simulink model "DoorWinBltSigMgr"  (R2022b)
settings: Solver=FixedStepDiscrete  StartTime=0.0  StopTime=10.0  FixedStep=0.1  RelTol=1e-3
settings Model: Creator=blue.ge  LastModifiedBy=blue.ge
settings DataIOCC: SaveFormat=StructureWithTime
settings OptimizationCC: DefaultParameterBehavior=Inlined  EnhancedBackFolding=on  PassReuseOutputArgsAs=Individual arguments  ZeroExternalMemoryAtStartup=off  ZeroInternalMemoryAtStartup=off  OptimizeBlockOrder=speed  DifferentSizesBufferReuse=on  OptimizationCustomize=off  EfficientTunableParamExpr=on
settings DebuggingCC: ParameterTunabilityLossMsg=error
settings ModelReferenceCC: ModelReferenceNumInstancesAllowed=Single
settings SFSimCC: MATLABDynamicMemAlloc=off
settings RTWCC: Description=AUTOSAR  SystemTargetFile=autosar.tlc  GenCodeOnly=on  TemplateMakefile=ert_default_tmf
settings CodeAppCC: ForceParamTrailComments=on  IgnoreCustomStorageClasses=off  OperatorAnnotations=on  SimulinkDataObjDesc=on  SFDataObjDesc=on  InsertBlockDesc=on
settings STFCustomTargetCC: TargetLangStandard=C99 (ISO)  MultiwordLength=256  ParMdlRefBuildCompliant=on  CompOptLevelCompliant=on  IncludeMdlTerminateFcn=off  CombineOutputUpdateFcns=on  SuppressErrorStatus=on  ERTCustomFileBanners=on  SupportNonFinite=off  SupportComplex=off  SupportContinuousTime=off  SupportNonInlinedSFcns=off  RemoveResetFunc=on  MATLABClassNameForMDSCustomization=Simulink.SoftwareTarget.ERTCustomization  AutosarCompliant=on  UseToolchainInfoCompliant=on  LookupTableObjectStructAxisOrder=2,1,3,4,...  LUTObjectStructOrderEvenSpacing=Size,Table,Breakpoints  SystemTargetFile=autosar.tlc
settings ERTTargetCC: TargetLangStandard=C99 (ISO)  ConcurrentExecutionCompliant=off  IncludeMdlTerminateFcn=off  SuppressErrorStatus=on  SupportNonFinite=off  SupportComplex=off  ModelStepFunctionPrototypeControlCompliant=off  CPPClassGenCompliant=off  AutosarCompliant=on  LookupTableObjectStructAxisOrder=2,1,3,4,...  LUTObjectStructOrderEvenSpacing=Size,Table,Breakpoints  InlinedParameterPlacement=NonHierarchical  ERTCustomFileTemplate=example_file_process.tlc

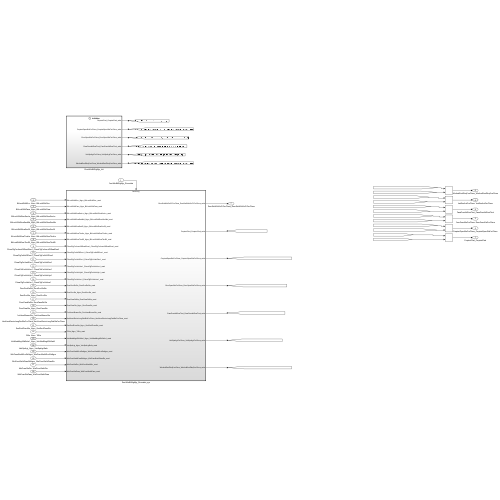
[thumbnail: Simulink's stored preview of the top-level diagram]
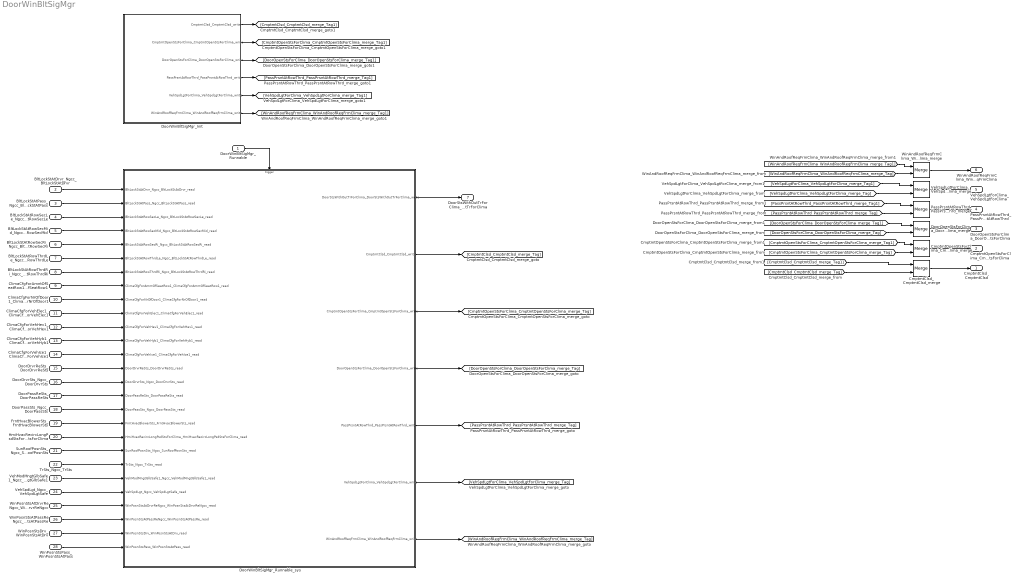
== system DoorWinBltSigMgr ==
  DoorWinBltSigMgr_Runnable : Inport  SampleTime=0.1  OutputFunctionCall=on
  BltLockStAtDrvr_Ngcc_BltLockStAtDrvr : Inport  Port=2  OutDataTypeStr=Bus: BltLockStFrnt  PortDimensions=1  BusOutputAsStruct=on
  BltLockStAtPass_Ngcc_BltLockStAtPass : Inport  Port=3  OutDataTypeStr=Bus: BltLockStFrnt  PortDimensions=1  BusOutputAsStruct=on
  BltLockStAtRowSecLe_Ngcc_BltLockStAtRowSecLe : Inport  Port=4  OutDataTypeStr=Bus: BltLockStRe  PortDimensions=1  BusOutputAsStruct=on
  BltLockStAtRowSecMid_Ngcc_BltLockStAtRowSecMid : Inport  Port=5  OutDataTypeStr=Bus: BltLockStRe  PortDimensions=1  BusOutputAsStruct=on
  BltLockStAtRowSecRi_Ngcc_BltLockStAtRowSecRi : Inport  Port=6  OutDataTypeStr=Bus: BltLockStRe  PortDimensions=1  BusOutputAsStruct=on
  BltLockStAtRowThrdLe_Ngcc_BltLockStAtRowThrdLe : Inport  Port=7  OutDataTypeStr=Bus: BltLockStRe  PortDimensions=1  BusOutputAsStruct=on
  BltLockStAtRowThrdRi_Ngcc_BltLockStAtRowThrdRi : Inport  Port=8  OutDataTypeStr=Bus: BltLockStRe  PortDimensions=1  BusOutputAsStruct=on
  ClimaCfgForAmntOfSeatRow1_ClimaCfgForAmntOfSeatRow1 : Inport  Port=9  OutDataTypeStr=Enum: ClimaCfgForAmntOfSeatRow1  PortDimensions=1
  ClimaCfgForNrOfDoor1_ClimaCfgForNrOfDoor1 : Inport  Port=10  OutDataTypeStr=Enum: ClimaCfgForNrOfDoor1  PortDimensions=1
  ClimaCfgForVehElec1_ClimaCfgForVehElec1 : Inport  Port=11  OutDataTypeStr=Boolean  PortDimensions=1
  ClimaCfgForVehHev1_ClimaCfgForVehHev1 : Inport  Port=12  OutDataTypeStr=Boolean  PortDimensions=1
  ClimaCfgForVehHyb1_ClimaCfgForVehHyb1 : Inport  Port=13  OutDataTypeStr=Boolean  PortDimensions=1
  ClimaCfgForVehIce1_ClimaCfgForVehIce1 : Inport  Port=14  OutDataTypeStr=Boolean  PortDimensions=1
  DoorDrvrReSts_DoorDrvrReSts : Inport  Port=15  OutDataTypeStr=Enum: DoorSts2  PortDimensions=1
  DoorDrvrSts_Ngcc_DoorDrvrSts : Inport  Port=16  OutDataTypeStr=Enum: DoorSts2  PortDimensions=1
  DoorPassReSts_DoorPassReSts : Inport  Port=17  OutDataTypeStr=Enum: DoorSts2  PortDimensions=1
  DoorPassSts_Ngcc_DoorPassSts : Inport  Port=18  OutDataTypeStr=Enum: DoorSts2  PortDimensions=1
  FrntHvacBlowerSts_FrntHvacBlowerSts : Inport  Port=19  OutDataTypeStr=Enum: HvacFanSts  PortDimensions=1
  HmiHvacRecircLongPsdStsForClima_HmiHvacRecircLongPsdStsForClima : Inport  Port=20  OutDataTypeStr=Enum: OnOff1  PortDimensions=1
  SunRoofPosnSts_Ngcc_SunRoofPosnSts : Inport  Port=21  OutDataTypeStr=Enum: WinAndRoofAndCurtPosnTyp  PortDimensions=1
  TrSts_Ngcc_TrSts : Inport  Port=22  OutDataTypeStr=Enum: DoorSts2  PortDimensions=1
  VehModMngtGlbSafe1_Ngcc_VehModMngtGlbSafe1 : Inport  Port=23  OutDataTypeStr=Bus: VehModMngtGlbSafe1  PortDimensions=1  BusOutputAsStruct=on
  VehSpdLgt_Ngcc_VehSpdLgtSafe : Inport  Port=24  OutDataTypeStr=Bus: VehSpdLgtSafe1  PortDimensions=1  BusOutputAsStruct=on
  WinPosnStsAtDrvrReNgcc_WinPosnStsAtDrvrReNgcc : Inport  Port=25  OutDataTypeStr=Enum: WinAndRoofAndCurtPosnTyp  PortDimensions=1
  WinPosnStsAtPassReNgcc_WinPosnStsAtPassRe : Inport  Port=26  OutDataTypeStr=Enum: WinAndRoofAndCurtPosnTyp  PortDimensions=1
  WinPosnStsDrv_WinPosnStsAtDrv : Inport  Port=27  OutDataTypeStr=Enum: WinAndRoofAndCurtPosnTyp  PortDimensions=1
  WinPosnStsPass_WinPosnStsAtPass : Inport  Port=28  OutDataTypeStr=Enum: WinAndRoofAndCurtPosnTyp  PortDimensions=1
  CmptmtClsd_CmptmtClsd_merge : Merge
  CmptmtOpenStsForClima_CmptmtOpenStsForClima_merge : Merge
  DoorOpenStsForClima_DoorOpenStsForClima_merge : Merge
  DoorWinBltSigMgr_Init : Subsystem
  DoorWinBltSigMgr_Runnable_sys : Subsystem
  PassPrsntAtRowThrd_PassPrsntAtRowThrd_merge : Merge
  VehSpdLgtForClima_VehSpdLgtForClima_merge : Merge
  WinAndRoofReqFrmClima_WinAndRoofReqFrmClima_merge : Merge
  CmptmtClsd_CmptmtClsd : Outport  OutDataTypeStr=Boolean  PortDimensions=1
  CmptmtOpenStsForClima_CmptmtOpenStsForClima : Outport  Port=2  OutDataTypeStr=Boolean  PortDimensions=1
  DoorOpenStsForClima_DoorOpenStsForClima : Outport  Port=3  OutDataTypeStr=Boolean  PortDimensions=1
  PassPrsntAtRowThrd_PassPrsntAtRowThrd : Outport  Port=4  OutDataTypeStr=Boolean  PortDimensions=1
  VehSpdLgtForClima_VehSpdLgtForClima : Outport  Port=5  OutDataTypeStr=Bus: VehSpdLgtForClima  PortDimensions=1  BusOutputAsStruct=on
  WinAndRoofReqFrmClima_WinAndRoofReqFrmClima : Outport  Port=6  OutDataTypeStr=Enum: WinOpenClsGlbSts  PortDimensions=1
  DoorStsWithOutTrForClima_DoorStsWithOutTrForClima : Outport  Port=7  OutDataTypeStr=Boolean  PortDimensions=1
  wires:
  BltLockStAtDrvr_Ngcc_BltLockStAtDrvr/1 -> DoorWinBltSigMgr_Runnable_sys/1(BltLockStAtDrvr_Ngcc_BltLockStAtDrvr_read)
  BltLockStAtPass_Ngcc_BltLockStAtPass/1 -> DoorWinBltSigMgr_Runnable_sys/2(BltLockStAtPass_Ngcc_BltLockStAtPass_read)
  BltLockStAtRowSecLe_Ngcc_BltLockStAtRowSecLe/1 -> DoorWinBltSigMgr_Runnable_sys/3(BltLockStAtRowSecLe_Ngcc_BltLockStAtRowSecLe_read)
  BltLockStAtRowSecMid_Ngcc_BltLockStAtRowSecMid/1 -> DoorWinBltSigMgr_Runnable_sys/4(BltLockStAtRowSecMid_Ngcc_BltLockStAtRowSecMid_read)
  BltLockStAtRowSecRi_Ngcc_BltLockStAtRowSecRi/1 -> DoorWinBltSigMgr_Runnable_sys/5(BltLockStAtRowSecRi_Ngcc_BltLockStAtRowSecRi_read)
  BltLockStAtRowThrdLe_Ngcc_BltLockStAtRowThrdLe/1 -> DoorWinBltSigMgr_Runnable_sys/6(BltLockStAtRowThrdLe_Ngcc_BltLockStAtRowThrdLe_read)
  BltLockStAtRowThrdRi_Ngcc_BltLockStAtRowThrdRi/1 -> DoorWinBltSigMgr_Runnable_sys/7(BltLockStAtRowThrdRi_Ngcc_BltLockStAtRowThrdRi_read)
  ClimaCfgForAmntOfSeatRow1_ClimaCfgForAmntOfSeatRow1/1 -> DoorWinBltSigMgr_Runnable_sys/8(ClimaCfgForAmntOfSeatRow1_ClimaCfgForAmntOfSeatRow1_read)
  ClimaCfgForNrOfDoor1_ClimaCfgForNrOfDoor1/1 -> DoorWinBltSigMgr_Runnable_sys/9(ClimaCfgForNrOfDoor1_ClimaCfgForNrOfDoor1_read)
  ClimaCfgForVehElec1_ClimaCfgForVehElec1/1 -> DoorWinBltSigMgr_Runnable_sys/10(ClimaCfgForVehElec1_ClimaCfgForVehElec1_read)
  ClimaCfgForVehHev1_ClimaCfgForVehHev1/1 -> DoorWinBltSigMgr_Runnable_sys/11(ClimaCfgForVehHev1_ClimaCfgForVehHev1_read)
  ClimaCfgForVehHyb1_ClimaCfgForVehHyb1/1 -> DoorWinBltSigMgr_Runnable_sys/12(ClimaCfgForVehHyb1_ClimaCfgForVehHyb1_read)
  ClimaCfgForVehIce1_ClimaCfgForVehIce1/1 -> DoorWinBltSigMgr_Runnable_sys/13(ClimaCfgForVehIce1_ClimaCfgForVehIce1_read)
  DoorDrvrReSts_DoorDrvrReSts/1 -> DoorWinBltSigMgr_Runnable_sys/14(DoorDrvrReSts_DoorDrvrReSts_read)
  DoorDrvrSts_Ngcc_DoorDrvrSts/1 -> DoorWinBltSigMgr_Runnable_sys/15(DoorDrvrSts_Ngcc_DoorDrvrSts_read)
  DoorPassReSts_DoorPassReSts/1 -> DoorWinBltSigMgr_Runnable_sys/16(DoorPassReSts_DoorPassReSts_read)
  DoorPassSts_Ngcc_DoorPassSts/1 -> DoorWinBltSigMgr_Runnable_sys/17(DoorPassSts_Ngcc_DoorPassSts_read)
  FrntHvacBlowerSts_FrntHvacBlowerSts/1 -> DoorWinBltSigMgr_Runnable_sys/18(FrntHvacBlowerSts_FrntHvacBlowerSts_read)
  HmiHvacRecircLongPsdStsForClima_HmiHvacRecircLongPsdStsForClima/1 -> DoorWinBltSigMgr_Runnable_sys/19(HmiHvacRecircLongPsdStsForClima_HmiHvacRecircLongPsdStsForClima_read)
  SunRoofPosnSts_Ngcc_SunRoofPosnSts/1 -> DoorWinBltSigMgr_Runnable_sys/20(SunRoofPosnSts_Ngcc_SunRoofPosnSts_read)
  TrSts_Ngcc_TrSts/1 -> DoorWinBltSigMgr_Runnable_sys/21(TrSts_Ngcc_TrSts_read)
  VehModMngtGlbSafe1_Ngcc_VehModMngtGlbSafe1/1 -> DoorWinBltSigMgr_Runnable_sys/22(VehModMngtGlbSafe1_Ngcc_VehModMngtGlbSafe1_read)
  VehSpdLgt_Ngcc_VehSpdLgtSafe/1 -> DoorWinBltSigMgr_Runnable_sys/23(VehSpdLgt_Ngcc_VehSpdLgtSafe_read)
  WinPosnStsAtDrvrReNgcc_WinPosnStsAtDrvrReNgcc/1 -> DoorWinBltSigMgr_Runnable_sys/24(WinPosnStsAtDrvrReNgcc_WinPosnStsAtDrvrReNgcc_read)
  WinPosnStsAtPassReNgcc_WinPosnStsAtPassRe/1 -> DoorWinBltSigMgr_Runnable_sys/25(WinPosnStsAtPassReNgcc_WinPosnStsAtPassRe_read)
  WinPosnStsDrv_WinPosnStsAtDrv/1 -> DoorWinBltSigMgr_Runnable_sys/26(WinPosnStsDrv_WinPosnStsAtDrv_read)
  WinPosnStsPass_WinPosnStsAtPass/1 -> DoorWinBltSigMgr_Runnable_sys/27(WinPosnStsPass_WinPosnStsAtPass_read)
  DoorWinBltSigMgr_Runnable_sys/1(DoorStsWithOutTrForClima_DoorStsWithOutTrForClima_write) -> DoorStsWithOutTrForClima_DoorStsWithOutTrForClima/1
  CmptmtClsd_CmptmtClsd_merge/1 -> CmptmtClsd_CmptmtClsd/1
  CmptmtOpenStsForClima_CmptmtOpenStsForClima_merge/1 -> CmptmtOpenStsForClima_CmptmtOpenStsForClima/1
  DoorOpenStsForClima_DoorOpenStsForClima_merge/1 -> DoorOpenStsForClima_DoorOpenStsForClima/1
  PassPrsntAtRowThrd_PassPrsntAtRowThrd_merge/1 -> PassPrsntAtRowThrd_PassPrsntAtRowThrd/1
  VehSpdLgtForClima_VehSpdLgtForClima_merge/1 -> VehSpdLgtForClima_VehSpdLgtForClima/1
  WinAndRoofReqFrmClima_WinAndRoofReqFrmClima_merge/1 -> WinAndRoofReqFrmClima_WinAndRoofReqFrmClima/1
  DoorWinBltSigMgr_Runnable_sys/7(WinAndRoofReqFrmClima_WinAndRoofReqFrmClima_write) -> [WinAndRoofReqFrmClima_WinAndRoofReqFrmClima_merge_Tag]
  [WinAndRoofReqFrmClima_WinAndRoofReqFrmClima_merge_Tag] -> WinAndRoofReqFrmClima_WinAndRoofReqFrmClima_merge/2
  DoorWinBltSigMgr_Init/6(WinAndRoofReqFrmClima_WinAndRoofReqFrmClima_write) -> [WinAndRoofReqFrmClima_WinAndRoofReqFrmClima_merge_Tag1]
  [WinAndRoofReqFrmClima_WinAndRoofReqFrmClima_merge_Tag1] -> WinAndRoofReqFrmClima_WinAndRoofReqFrmClima_merge/1
  DoorWinBltSigMgr_Runnable_sys/6(VehSpdLgtForClima_VehSpdLgtForClima_write) -> [VehSpdLgtForClima_VehSpdLgtForClima_merge_Tag]
  [VehSpdLgtForClima_VehSpdLgtForClima_merge_Tag] -> VehSpdLgtForClima_VehSpdLgtForClima_merge/2
  DoorWinBltSigMgr_Init/5(VehSpdLgtForClima_VehSpdLgtForClima_write) -> [VehSpdLgtForClima_VehSpdLgtForClima_merge_Tag1]
  [VehSpdLgtForClima_VehSpdLgtForClima_merge_Tag1] -> VehSpdLgtForClima_VehSpdLgtForClima_merge/1
  DoorWinBltSigMgr_Runnable_sys/5(PassPrsntAtRowThrd_PassPrsntAtRowThrd_write) -> [PassPrsntAtRowThrd_PassPrsntAtRowThrd_merge_Tag]
  [PassPrsntAtRowThrd_PassPrsntAtRowThrd_merge_Tag] -> PassPrsntAtRowThrd_PassPrsntAtRowThrd_merge/2
  DoorWinBltSigMgr_Init/4(PassPrsntAtRowThrd_PassPrsntAtRowThrd_write) -> [PassPrsntAtRowThrd_PassPrsntAtRowThrd_merge_Tag1]
  [PassPrsntAtRowThrd_PassPrsntAtRowThrd_merge_Tag1] -> PassPrsntAtRowThrd_PassPrsntAtRowThrd_merge/1
  DoorWinBltSigMgr_Runnable_sys/4(DoorOpenStsForClima_DoorOpenStsForClima_write) -> [DoorOpenStsForClima_DoorOpenStsForClima_merge_Tag]
  [DoorOpenStsForClima_DoorOpenStsForClima_merge_Tag] -> DoorOpenStsForClima_DoorOpenStsForClima_merge/2
  DoorWinBltSigMgr_Init/3(DoorOpenStsForClima_DoorOpenStsForClima_write) -> [DoorOpenStsForClima_DoorOpenStsForClima_merge_Tag1]
  [DoorOpenStsForClima_DoorOpenStsForClima_merge_Tag1] -> DoorOpenStsForClima_DoorOpenStsForClima_merge/1
  DoorWinBltSigMgr_Runnable_sys/3(CmptmtOpenStsForClima_CmptmtOpenStsForClima_write) -> [CmptmtOpenStsForClima_CmptmtOpenStsForClima_merge_Tag]
  [CmptmtOpenStsForClima_CmptmtOpenStsForClima_merge_Tag] -> CmptmtOpenStsForClima_CmptmtOpenStsForClima_merge/2
  DoorWinBltSigMgr_Init/2(CmptmtOpenStsForClima_CmptmtOpenStsForClima_write) -> [CmptmtOpenStsForClima_CmptmtOpenStsForClima_merge_Tag1]
  [CmptmtOpenStsForClima_CmptmtOpenStsForClima_merge_Tag1] -> CmptmtOpenStsForClima_CmptmtOpenStsForClima_merge/1
  DoorWinBltSigMgr_Runnable_sys/2(CmptmtClsd_CmptmtClsd_write) -> [CmptmtClsd_CmptmtClsd_merge_Tag]
  [CmptmtClsd_CmptmtClsd_merge_Tag] -> CmptmtClsd_CmptmtClsd_merge/2
  DoorWinBltSigMgr_Init/1(CmptmtClsd_CmptmtClsd_write) -> [CmptmtClsd_CmptmtClsd_merge_Tag1]
  [CmptmtClsd_CmptmtClsd_merge_Tag1] -> CmptmtClsd_CmptmtClsd_merge/1
  DoorWinBltSigMgr_Runnable/1 -> DoorWinBltSigMgr_Runnable_sys/Trigger

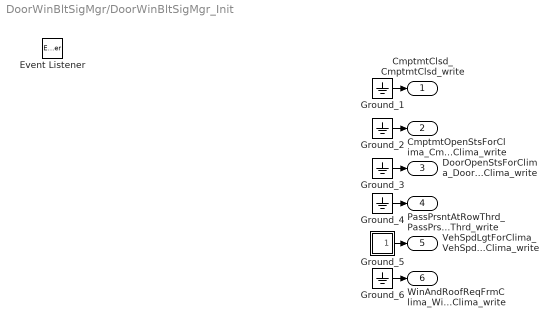
== system DoorWinBltSigMgr/DoorWinBltSigMgr_Init ==
  Event Listener : EventListener  EventName=reset
  Ground_{1..4,6} (5 alike) : Ground
  Ground_5 : Subsystem
  CmptmtClsd_CmptmtClsd_write : Outport
  CmptmtOpenStsForClima_CmptmtOpenStsForClima_write : Outport  Port=2
  DoorOpenStsForClima_DoorOpenStsForClima_write : Outport  Port=3
  PassPrsntAtRowThrd_PassPrsntAtRowThrd_write : Outport  Port=4
  VehSpdLgtForClima_VehSpdLgtForClima_write : Outport  Port=5
  WinAndRoofReqFrmClima_WinAndRoofReqFrmClima_write : Outport  Port=6
  wires:
  Ground_1/1 -> CmptmtClsd_CmptmtClsd_write/1
  Ground_2/1 -> CmptmtOpenStsForClima_CmptmtOpenStsForClima_write/1
  Ground_3/1 -> DoorOpenStsForClima_DoorOpenStsForClima_write/1
  Ground_4/1 -> PassPrsntAtRowThrd_PassPrsntAtRowThrd_write/1
  Ground_5/1(Out1) -> VehSpdLgtForClima_VehSpdLgtForClima_write/1
  Ground_6/1 -> WinAndRoofReqFrmClima_WinAndRoofReqFrmClima_write/1

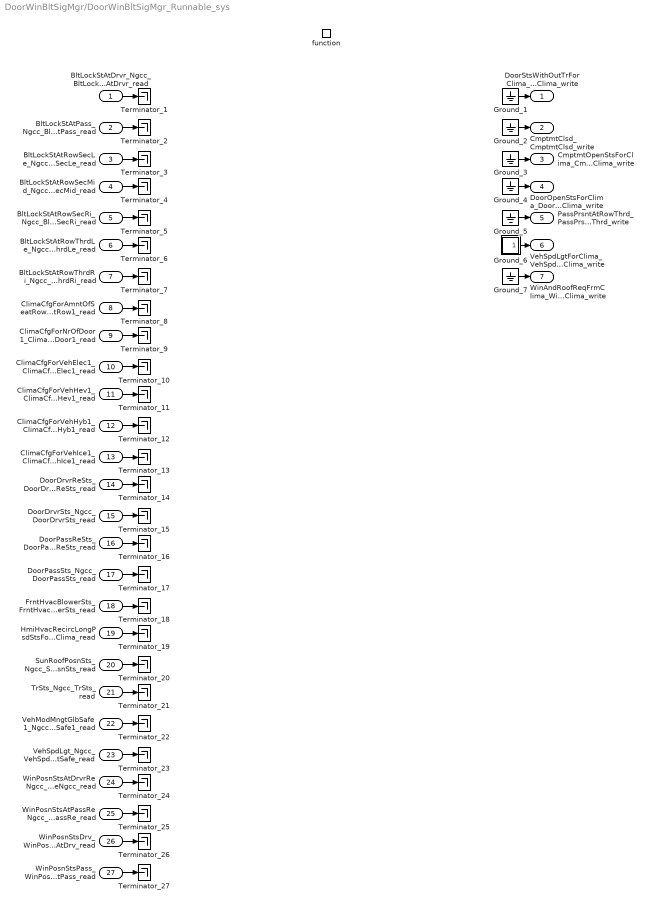
== system DoorWinBltSigMgr/DoorWinBltSigMgr_Runnable_sys ==
  BltLockStAtDrvr_Ngcc_BltLockStAtDrvr_read : Inport
  BltLockStAtPass_Ngcc_BltLockStAtPass_read : Inport  Port=2
  BltLockStAtRowSecLe_Ngcc_BltLockStAtRowSecLe_read : Inport  Port=3
  BltLockStAtRowSecMid_Ngcc_BltLockStAtRowSecMid_read : Inport  Port=4
  BltLockStAtRowSecRi_Ngcc_BltLockStAtRowSecRi_read : Inport  Port=5
  BltLockStAtRowThrdLe_Ngcc_BltLockStAtRowThrdLe_read : Inport  Port=6
  BltLockStAtRowThrdRi_Ngcc_BltLockStAtRowThrdRi_read : Inport  Port=7
  ClimaCfgForAmntOfSeatRow1_ClimaCfgForAmntOfSeatRow1_read : Inport  Port=8
  ClimaCfgForNrOfDoor1_ClimaCfgForNrOfDoor1_read : Inport  Port=9
  ClimaCfgForVehElec1_ClimaCfgForVehElec1_read : Inport  Port=10
  ClimaCfgForVehHev1_ClimaCfgForVehHev1_read : Inport  Port=11
  ClimaCfgForVehHyb1_ClimaCfgForVehHyb1_read : Inport  Port=12
  ClimaCfgForVehIce1_ClimaCfgForVehIce1_read : Inport  Port=13
  DoorDrvrReSts_DoorDrvrReSts_read : Inport  Port=14
  DoorDrvrSts_Ngcc_DoorDrvrSts_read : Inport  Port=15
  DoorPassReSts_DoorPassReSts_read : Inport  Port=16
  DoorPassSts_Ngcc_DoorPassSts_read : Inport  Port=17
  FrntHvacBlowerSts_FrntHvacBlowerSts_read : Inport  Port=18
  HmiHvacRecircLongPsdStsForClima_HmiHvacRecircLongPsdStsForClima_read : Inport  Port=19
  SunRoofPosnSts_Ngcc_SunRoofPosnSts_read : Inport  Port=20
  TrSts_Ngcc_TrSts_read : Inport  Port=21
  VehModMngtGlbSafe1_Ngcc_VehModMngtGlbSafe1_read : Inport  Port=22
  VehSpdLgt_Ngcc_VehSpdLgtSafe_read : Inport  Port=23
  WinPosnStsAtDrvrReNgcc_WinPosnStsAtDrvrReNgcc_read : Inport  Port=24
  WinPosnStsAtPassReNgcc_WinPosnStsAtPassRe_read : Inport  Port=25
  WinPosnStsDrv_WinPosnStsAtDrv_read : Inport  Port=26
  WinPosnStsPass_WinPosnStsAtPass_read : Inport  Port=27
  function : TriggerPort  TriggerType=function-call  VariantControl=(inherit)  SampleTimeType=periodic  SampleTime=-1
  Ground_{1..5,7} (6 alike) : Ground
  Ground_6 : Subsystem
  Terminator_{1..27} (27 alike) : Terminator
  DoorStsWithOutTrForClima_DoorStsWithOutTrForClima_write : Outport
      settings: EnsureOutportIsVirtual=on
  CmptmtClsd_CmptmtClsd_write : Outport  Port=2
      settings: EnsureOutportIsVirtual=on
  CmptmtOpenStsForClima_CmptmtOpenStsForClima_write : Outport  Port=3
      settings: EnsureOutportIsVirtual=on
  DoorOpenStsForClima_DoorOpenStsForClima_write : Outport  Port=4
      settings: EnsureOutportIsVirtual=on
  PassPrsntAtRowThrd_PassPrsntAtRowThrd_write : Outport  Port=5
      settings: EnsureOutportIsVirtual=on
  VehSpdLgtForClima_VehSpdLgtForClima_write : Outport  Port=6
      settings: EnsureOutportIsVirtual=on
  WinAndRoofReqFrmClima_WinAndRoofReqFrmClima_write : Outport  Port=7
      settings: EnsureOutportIsVirtual=on
  wires:
  BltLockStAtDrvr_Ngcc_BltLockStAtDrvr_read/1 -> Terminator_1/1
  BltLockStAtPass_Ngcc_BltLockStAtPass_read/1 -> Terminator_2/1
  BltLockStAtRowSecLe_Ngcc_BltLockStAtRowSecLe_read/1 -> Terminator_3/1
  BltLockStAtRowSecMid_Ngcc_BltLockStAtRowSecMid_read/1 -> Terminator_4/1
  BltLockStAtRowSecRi_Ngcc_BltLockStAtRowSecRi_read/1 -> Terminator_5/1
  BltLockStAtRowThrdLe_Ngcc_BltLockStAtRowThrdLe_read/1 -> Terminator_6/1
  BltLockStAtRowThrdRi_Ngcc_BltLockStAtRowThrdRi_read/1 -> Terminator_7/1
  ClimaCfgForAmntOfSeatRow1_ClimaCfgForAmntOfSeatRow1_read/1 -> Terminator_8/1
  ClimaCfgForNrOfDoor1_ClimaCfgForNrOfDoor1_read/1 -> Terminator_9/1
  ClimaCfgForVehElec1_ClimaCfgForVehElec1_read/1 -> Terminator_10/1
  ClimaCfgForVehHev1_ClimaCfgForVehHev1_read/1 -> Terminator_11/1
  ClimaCfgForVehHyb1_ClimaCfgForVehHyb1_read/1 -> Terminator_12/1
  ClimaCfgForVehIce1_ClimaCfgForVehIce1_read/1 -> Terminator_13/1
  DoorDrvrReSts_DoorDrvrReSts_read/1 -> Terminator_14/1
  DoorDrvrSts_Ngcc_DoorDrvrSts_read/1 -> Terminator_15/1
  DoorPassReSts_DoorPassReSts_read/1 -> Terminator_16/1
  DoorPassSts_Ngcc_DoorPassSts_read/1 -> Terminator_17/1
  FrntHvacBlowerSts_FrntHvacBlowerSts_read/1 -> Terminator_18/1
  HmiHvacRecircLongPsdStsForClima_HmiHvacRecircLongPsdStsForClima_read/1 -> Terminator_19/1
  SunRoofPosnSts_Ngcc_SunRoofPosnSts_read/1 -> Terminator_20/1
  TrSts_Ngcc_TrSts_read/1 -> Terminator_21/1
  VehModMngtGlbSafe1_Ngcc_VehModMngtGlbSafe1_read/1 -> Terminator_22/1
  VehSpdLgt_Ngcc_VehSpdLgtSafe_read/1 -> Terminator_23/1
  WinPosnStsAtDrvrReNgcc_WinPosnStsAtDrvrReNgcc_read/1 -> Terminator_24/1
  WinPosnStsAtPassReNgcc_WinPosnStsAtPassRe_read/1 -> Terminator_25/1
  WinPosnStsDrv_WinPosnStsAtDrv_read/1 -> Terminator_26/1
  WinPosnStsPass_WinPosnStsAtPass_read/1 -> Terminator_27/1
  Ground_1/1 -> DoorStsWithOutTrForClima_DoorStsWithOutTrForClima_write/1
  Ground_2/1 -> CmptmtClsd_CmptmtClsd_write/1
  Ground_3/1 -> CmptmtOpenStsForClima_CmptmtOpenStsForClima_write/1
  Ground_4/1 -> DoorOpenStsForClima_DoorOpenStsForClima_write/1
  Ground_5/1 -> PassPrsntAtRowThrd_PassPrsntAtRowThrd_write/1
  Ground_6/1(Out1) -> VehSpdLgtForClima_VehSpdLgtForClima_write/1
  Ground_7/1 -> WinAndRoofReqFrmClima_WinAndRoofReqFrmClima_write/1

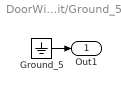
== system DoorWinBltSigMgr/DoorWinBltSigMgr_Init/Ground_5 ==
  Ground_5 : Ground
  Out1 : Outport  OutDataTypeStr=Bus: VehSpdLgtForClima  PortDimensions=1
  wires:
  Ground_5/1 -> Out1/1

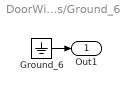
== system DoorWinBltSigMgr/DoorWinBltSigMgr_Runnable_sys/Ground_6 ==
  Ground_6 : Ground
  Out1 : Outport  OutDataTypeStr=Bus: VehSpdLgtForClima  PortDimensions=1
  wires:
  Ground_6/1 -> Out1/1
